simulink model "controller_observer_AllAgents_v5"  (Simulink 8.4)
settings: Solver=ode1  StartTime=0.0  StopTime=inf  FixedStep=60*1  RelTol=1e-3
values from neighbouring files (symbols this model uses):
  Cpw = 4180    [readepJSONout.m]
  m_max = 3    [DesignParameters.m]
  mc_MAX = 2    [DesignParameters.m]
  Tamb_additive_Sensor_fault_time = 60*60*24*(Tamb_day_ofthe_add_fault + Tamb_time_ofthe_add_fault)    (= 129600)    [faults_setting.m]
  Tamb_additive_Sensor_fault_value = 10    [faults_setting.m]
  Tamb_mult_Sensor_fault_time = 60*60*24*(Tamb_day_ofthe_mult_fault + Tamb_time_ofthe_mult_fault)    (= 129600)    [faults_setting.m]
  Tamb_mult_Sensor_fault_value = 1.1    [faults_setting.m]
  Ts = 3600/dat.Timestep.Timestep_0x20_1.number_of_timesteps_per_hour    [readepJSONout.m]
  Tst_C_additive_Sensor_fault_time = 60*60*24*(Tst_C_day_ofthe_add_fault + Tst_C_time_ofthe_add_fault)    (= 129600)    [faults_setting.m]
  Tst_C_additive_Sensor_fault_value = 10    [faults_setting.m]
  Tst_C_mult_Sensor_fault_time = 60*60*24*(Tst_C_day_ofthe_mult_fault + Tst_C_time_ofthe_mult_fault)    (= 129600)    [faults_setting.m]
  Tst_C_mult_Sensor_fault_value = 1.1    [faults_setting.m]
  Tst_H_additive_Sensor_fault_time = 60*60*24*(Tst_H_day_ofthe_add_fault + Tst_H_time_ofthe_add_fault)    (= 129600)    [faults_setting.m]
  Tst_H_additive_Sensor_fault_value = 10    [faults_setting.m]
  Tst_H_mult_Sensor_fault_time = 60*60*24*(Tst_H_day_ofthe_mult_fault + Tst_H_time_ofthe_mult_fault)    (= 129600)    [faults_setting.m]
  Tst_H_mult_Sensor_fault_value = 1.1    [faults_setting.m]
undefined symbols (not defined in the file or in its folder): building, COP, Ehp_max, fsa_E, fsa_ET, fsa_N, fsa_NT, fsa_W, fsa_WT, h_E, h_ET, h_N, h_NT, h_W, h_WT, K_NtoNT, K_NTtoN, Ke_E, Ke_ET, Ke_N, Ke_NT, Ke_WT, Km_E, Km_ET, Km_N, Km_NT, Km_W, Km_WT, Kout_N, Kout_NT, Kout_W, Kout_WT, Kst, Ksta, Kstcij, KstRef, Kw_E, Kw_ET, Kw_N, Kw_NT, Kw_W, Kw_WT
libraries: simulink x61
(part 10 of 36)

== system controller_observer_AllAgents_v5/Controller/CorridorPod3_Cooling ==
  In1 : Inport
  Enable : EnablePort
  Add : Sum  SampleTime=Ts
  Add1 : Sum  SampleTime=Ts
  CoilControlGains : Constant  Value=[building.zone(12).Kesa_C; building.zone(12).Ksa_C; building.zone(12).K_C; building.zone(12).Kma_C; building.zone(12).Kf_C]  SampleTime=Ts  FramePeriod=Ts
  Constant : Constant  Value=0.00001  SampleTime=Ts
  Constant1 : Constant  Value=0
  Demux : Demux  Outputs=[1 1 1 1 1 numel(building.zone(12).neighbour)]
  Discrete PID Controller : simulink/Continuous/PID Controller  Controller=PID  TimeDomain=Continuous-time  SampleTime=-1  IntegratorMethod=Forward Euler  FilterMethod=Forward Euler  Form=Parallel  P=1  I=1  D=0  N=100  InitialConditionSource=internal  InitialConditionForIntegrator=0  InitialConditionForFilter=0  ExternalReset=none  LimitOutput=off  UpperSaturationLimit=inf  LowerSaturationLimit=-inf  LinearizeAsGain=on  AntiWindupMode=none  Kb=1  TrackingMode=off  Kt=1  PParamMin=[]  PParamMax=[]  IParamMin=[]  IParamMax=[]  DParamMin=[]  DParamMax=[]  NParamMin=[]  NParamMax=[]  KbParamMin=[]  KbParamMax=[]  KtParamMin=[]  KtParamMax=[]  POutMin=[]  POutMax=[]  IOutMin=[]  IOutMax=[]  DOutMin=[]  DOutMax=[]  NOutMin=[]  NOutMax=[]  KbOutMin=[]  KbOutMax=[]  KtOutMin=[]  KtOutMax=[]  IntegratorOutMin=[]  IntegratorOutMax=[]  FilterOutMin=[]  FilterOutMax=[]  SumOutMin=[]  SumOutMax=[]  SumI1OutMin=[]  SumI1OutMax=[]  SumI2OutMin=[]  SumI2OutMax=[]  SumI3OutMin=[]  SumI3OutMax=[]  SumDOutMin=[]  SumDOutMax=[]  SaturationOutMin=[]  SaturationOutMax=[]  IntegratorContinuousStateAttributes=''  IntegratorStateMustResolveToSignalObject=off  IntegratorRTWStateStorageClass=Auto  FilterContinuousStateAttributes=''  FilterStateMustResolveToSignalObject=off  FilterRTWStateStorageClass=Auto  DifferentiatorICPrevScaledInput=0  DifferentiatorOutMin=[]  DifferentiatorOutMax=[]  UseFilter=on
  Divide : Product  Inputs=*/  SampleTime=Ts
  Divide1 : Product  Inputs=*/  SampleTime=Ts
  Dot Product : DotProduct  SampleTime=Ts  InputSameDT=on  OutDataTypeStr=Inherit: Inherit via internal rule
  Dot Product1 : DotProduct  SampleTime=Ts  InputSameDT=on  OutDataTypeStr=Inherit: Inherit via internal rule
  Dot Product2 : DotProduct  SampleTime=Ts  InputSameDT=on  OutDataTypeStr=Inherit: Inherit via internal rule
  Gain{,1..3} (4 alike) : Gain  Gain=-1  SampleTime=Ts
  If : If  IfExpression=u1 >= 0
  If Action Subsystem : Subsystem  TreatAsAtomicUnit=on
  If Action Subsystem1 : Subsystem  TreatAsAtomicUnit=on
  K_I_mat : ToFile  Filename=C:\Users\papad\Dropbox\Complete_building_simulator_Observers\Results_fz4_sensor\K_I_CorridorPod3.mat  SaveFormat=Timeseries
  MaxValveFlow : Constant  Value=m_max  SampleTime=Ts  FramePeriod=Ts
  Merge : Merge
  Mux : Mux  Inputs=3
  Mux1 : Mux  Inputs=2
  Mux2 : Mux  Inputs=5
  Mux3 : Mux  Inputs=5
      settings: DisplayOption=signals
  Product : Product  SampleTime=Ts
  Scope : Scope
  Scope1 : Scope
  Scope2 : Scope
  Subtract{,1..4} (5 alike) : Sum  Inputs=+-  SampleTime=Ts
  Tc_C_mat : ToFile  Filename=C:\Users\papad\Dropbox\Complete_building_simulator_Observers\Results_fz4_sensor\Tc_C_CorridorPod3.mat  SaveFormat=Timeseries
  Tc_C_ref_mat : ToFile  Filename=C:\Users\papad\Dropbox\Complete_building_simulator_Observers\Results_fz4_sensor\Tc_C_ref_CorridorPod3.mat  SaveFormat=Timeseries
  TsTc_err_C_mat : ToFile  Filename=C:\Users\papad\Dropbox\Complete_building_simulator_Observers\Results_fz4_sensor\TsTc_err_C_CorridorPod3.mat  SaveFormat=Timeseries
  Tsa_mat : ToFile  Filename=C:\Users\papad\Dropbox\Complete_building_simulator_Observers\Results_fz4_sensor\Tsa_CorridorPod3.mat  SaveFormat=Timeseries
  Tsa_ref_mat : ToFile  Filename=C:\Users\papad\Dropbox\Complete_building_simulator_Observers\Results_fz4_sensor\Tsa_ref_CorridorPod3.mat  SaveFormat=Timeseries
  Tst_Tc_C_mat : ToFile  Filename=C:\Users\papad\Dropbox\Complete_building_simulator_Observers\Results_fz4_sensor\Tst_Tc_C_CorridorPod3.mat  SaveFormat=Timeseries
  TzRef : Constant  Value=building.zone(12).TzRef  SampleTime=Ts  FramePeriod=Ts
  Tz_mat : ToFile  Filename=C:\Users\papad\Dropbox\Complete_building_simulator_Observers\Results_fz4_sensor\Tz_CorridorPod3.mat  SaveFormat=Timeseries
  Tz_ref_mat : ToFile  Filename=C:\Users\papad\Dropbox\Complete_building_simulator_Observers\Results_fz4_sensor\Tz_ref_CorridorPod3.mat  SaveFormat=Timeseries
  Unit Delay : UnitDelay  SampleTime=Ts
  ValveControlGains : Constant  Value=[building.zone(12).Kec_C; building.zone(12).Kc_C; building.zone(12).Kcsa_C]  SampleTime=Ts  FramePeriod=Ts
  ValveControlGains2 : Constant  SampleTime=Ts  FramePeriod=Ts
  ZoneControlGains : Constant  Value=[building.zone(12).Ke; building.zone(12).Kz; transpose(building.zone(12).Kij); building.zone(12).Kza; building.zone(12).hi]  SampleTime=Ts  FramePeriod=Ts
  er_Tz_mat : ToFile  Filename=C:\Users\papad\Dropbox\Complete_building_simulator_Observers\Results_fz4_sensor\er_Tz_CorridorPod3.mat  SaveFormat=Timeseries
  mc_C_mat : ToFile  Filename=C:\Users\papad\Dropbox\Complete_building_simulator_Observers\Results_fz4_sensor\mc_C_CorridorPod3.mat  SaveFormat=Timeseries
  mc_Tst_Tc_C_mat : ToFile  Filename=C:\Users\papad\Dropbox\Complete_building_simulator_Observers\Results_fz4_sensor\mc_Tst_Tc_C_CorridorPod3.mat  SaveFormat=Timeseries
  zone_gains_mat : ToFile  Filename=C:\Users\papad\Dropbox\Complete_building_simulator_Observers\Results_fz4_sensor\zone_gains_CorridorPod3.mat  SaveFormat=Timeseries
  Out1 : Outport
  Out2 : Outport  Port=2
  Out3 : Outport  Port=3
  wires:
  Mux/1 -> Dot Product/1
  CoilControlGains/1 -> Gain1/1
  Add/1 -> Unit Delay/1, K_I_mat/1, [K_I]
  Dot Product/1 -> Divide1/1, Scope/1, mc_Tst_Tc_C_mat/1, Divide/1
  Gain1/1 -> Dot Product1/2
  [TzRef] -> Subtract1/2
  ValveControlGains2/1 -> Mux2/5
  [Tst] -> Mux1/1, Subtract/1
  Mux2/1 -> Dot Product1/1
  Demux/6 -> [TzNeighbor]
  Demux/4 -> [Tsa]
  Mux3/1 -> Dot Product2/1
  Gain2/1 -> Dot Product2/2
  Subtract1/1 -> Add/1
  Dot Product2/1 -> Scope2/1, Tsa_ref_mat/1, [TsaRef], Subtract3/2, Mux2/2
  [Tc] -> Mux1/2, Subtract/2
  Dot Product1/1 -> Scope1/1, Tc_C_ref_mat/1, [TcRef], Mux/2, Subtract4/2
  [Tz] -> Subtract1/1
  Unit Delay/1 -> Add/2
  ZoneControlGains/1 -> zone_gains_mat/1, Gain2/1
  [Tsa] -> Mux/3
  [Tz] -> Mux2/3
  Demux/2 -> [Tamb]
  Demux/5 -> [Tst]
  Gain/1 -> Dot Product/2
  ValveControlGains/1 -> Gain/1
  In1/1 -> Demux/1
  [Tc] -> Add1/2
  Divide1/1 -> Add1/1
  MaxValveFlow/1 -> Divide1/2
  Add1/1 -> Out3/1, [TstRef]
  Product/1 -> Out2/1
  Gain3/1 -> Product/2
  Mux1/1 -> Tst_Tc_C_mat/1
  TzRef/1 -> [TzRef], Tz_ref_mat/1, Subtract2/2, Mux3/2
  Subtract3/1 -> Mux2/1
  [Tsa] -> Tsa_mat/1, Subtract3/1
  Subtract4/1 -> Mux/1
  [Tc] -> Subtract4/1, Tc_C_mat/1
  Constant1/1 -> If Action Subsystem/1(In1)
  If/1 -> If Action Subsystem/Action
  If/2 -> If Action Subsystem1/Action
  If Action Subsystem/1(Out1) -> Merge/1
  Subtract/1 -> TsTc_err_C_mat/1, Divide/2, If/1
  Divide/1 -> If Action Subsystem1/1(In1)
  If Action Subsystem1/1(Out1) -> Merge/2
  [Tz] -> Tz_mat/1, Subtract2/1
  [TzNeighbor] -> Mux3/3
  [Tamb] -> Mux2/4
  [Tamb] -> Mux3/4
  [K_I] -> Mux3/5
  Subtract2/1 -> Discrete PID Controller/1, er_Tz_mat/1, Mux3/1
  Demux/1 -> [Tz]
  Merge/1 -> mc_C_mat/1, Out1/1
  Demux/3 -> [Tc]

== system controller_observer_AllAgents_v5/Controller/CorridorPod3_Heating ==
  In1 : Inport
  Enable : EnablePort
  Add : Sum  SampleTime=Ts
  Add1 : Sum  SampleTime=Ts
  CoilControlGains : Constant  Value=[building.zone(12).Kesa_H; building.zone(12).Ksa_H; building.zone(12).K_H; building.zone(12).Kma_H; building.zone(12).Kf_H]  SampleTime=Ts  FramePeriod=Ts
  Constant : Constant  Value=0.00001  SampleTime=Ts
  Constant1 : Constant  Value=0
  Demux : Demux  Outputs=[1 1 1 1 1 numel(building.zone(12).neighbour)]
  Discrete PID Controller : simulink/Continuous/PID Controller  Controller=PID  TimeDomain=Continuous-time  SampleTime=-1  IntegratorMethod=Forward Euler  FilterMethod=Forward Euler  Form=Parallel  P=1  I=1  D=0  N=100  InitialConditionSource=internal  InitialConditionForIntegrator=0  InitialConditionForFilter=0  ExternalReset=none  LimitOutput=off  UpperSaturationLimit=inf  LowerSaturationLimit=-inf  LinearizeAsGain=on  AntiWindupMode=none  Kb=1  TrackingMode=off  Kt=1  PParamMin=[]  PParamMax=[]  IParamMin=[]  IParamMax=[]  DParamMin=[]  DParamMax=[]  NParamMin=[]  NParamMax=[]  KbParamMin=[]  KbParamMax=[]  KtParamMin=[]  KtParamMax=[]  POutMin=[]  POutMax=[]  IOutMin=[]  IOutMax=[]  DOutMin=[]  DOutMax=[]  NOutMin=[]  NOutMax=[]  KbOutMin=[]  KbOutMax=[]  KtOutMin=[]  KtOutMax=[]  IntegratorOutMin=[]  IntegratorOutMax=[]  FilterOutMin=[]  FilterOutMax=[]  SumOutMin=[]  SumOutMax=[]  SumI1OutMin=[]  SumI1OutMax=[]  SumI2OutMin=[]  SumI2OutMax=[]  SumI3OutMin=[]  SumI3OutMax=[]  SumDOutMin=[]  SumDOutMax=[]  SaturationOutMin=[]  SaturationOutMax=[]  IntegratorContinuousStateAttributes=''  IntegratorStateMustResolveToSignalObject=off  IntegratorRTWStateStorageClass=Auto  FilterContinuousStateAttributes=''  FilterStateMustResolveToSignalObject=off  FilterRTWStateStorageClass=Auto  DifferentiatorICPrevScaledInput=0  DifferentiatorOutMin=[]  DifferentiatorOutMax=[]  UseFilter=on
  Divide : Product  Inputs=*/  SampleTime=Ts
  Divide1 : Product  Inputs=*/  SampleTime=Ts
  Dot Product : DotProduct  SampleTime=Ts  InputSameDT=on  OutDataTypeStr=Inherit: Inherit via internal rule
  Dot Product1 : DotProduct  SampleTime=Ts  InputSameDT=on  OutDataTypeStr=Inherit: Inherit via internal rule
  Dot Product2 : DotProduct  SampleTime=Ts  InputSameDT=on  OutDataTypeStr=Inherit: Inherit via internal rule
  Gain{,1..3} (4 alike) : Gain  Gain=-1  SampleTime=Ts
  If : If  IfExpression=u1 <= 0
  If Action Subsystem : Subsystem  TreatAsAtomicUnit=on
  If Action Subsystem1 : Subsystem  TreatAsAtomicUnit=on
  MaxValveFlow : Constant  Value=m_max  SampleTime=Ts  FramePeriod=Ts
  Merge : Merge
  Mux : Mux  Inputs=3
  Mux1 : Mux  Inputs=2
  Mux2 : Mux  Inputs=5
  Mux3 : Mux  Inputs=5
      settings: DisplayOption=signals
  Product : Product  SampleTime=Ts
  Scope : Scope
  Scope1 : Scope
  Scope2 : Scope
  Subtract{,1..4} (5 alike) : Sum  Inputs=+-  SampleTime=Ts
  Tc_H_mat : ToFile  Filename=C:\Users\papad\Dropbox\Complete_building_simulator_Observers\Results_fz4_sensor\Tc_H_CorridorPod3.mat  SaveFormat=Timeseries
  Tc_H_ref_mat : ToFile  Filename=C:\Users\papad\Dropbox\Complete_building_simulator_Observers\Results_fz4_sensor\Tc_H_ref_CorridorPod3.mat  SaveFormat=Timeseries
  TsTc_err_H_mat : ToFile  Filename=C:\Users\papad\Dropbox\Complete_building_simulator_Observers\Results_fz4_sensor\TsTc_err_H_CorridorPod3.mat  SaveFormat=Timeseries
  Tst_Tc_H_mat : ToFile  Filename=C:\Users\papad\Dropbox\Complete_building_simulator_Observers\Results_fz4_sensor\Tst_Tc_H_CorridorPod3.mat  SaveFormat=Timeseries
  TzRef : Constant  Value=building.zone(12).TzRef  SampleTime=Ts  FramePeriod=Ts
  Unit Delay : UnitDelay  SampleTime=Ts
  ValveControlGains : Constant  Value=[building.zone(12).Kec_H; building.zone(12).Kc_H; building.zone(12).Kcsa_H]  SampleTime=Ts  FramePeriod=Ts
  ValveControlGains2 : Constant  SampleTime=Ts  FramePeriod=Ts
  ZoneControlGains : Constant  Value=[building.zone(12).Ke; building.zone(12).Kz; transpose(building.zone(12).Kij); building.zone(12).Kza; building.zone(12).hi]  SampleTime=Ts  FramePeriod=Ts
  mc_H_mat : ToFile  Filename=C:\Users\papad\Dropbox\Complete_building_simulator_Observers\Results_fz4_sensor\mc_H_CorridorPod3.mat  SaveFormat=Timeseries
  mc_Tst_Tc_H_mat : ToFile  Filename=C:\Users\papad\Dropbox\Complete_building_simulator_Observers\Results_fz4_sensor\mc_Tst_Tc_H_CorridorPod3.mat  SaveFormat=Timeseries
  Out1 : Outport
  Out2 : Outport  Port=2
  Out3 : Outport  Port=3
  wires:
  Mux/1 -> Dot Product/1
  CoilControlGains/1 -> Gain1/1
  Add/1 -> Unit Delay/1, [K_I]
  Dot Product/1 -> Divide1/1, Scope/1, mc_Tst_Tc_H_mat/1, Divide/1
  Gain1/1 -> Dot Product1/2
  [TzRef] -> Subtract1/2
  ValveControlGains2/1 -> Mux2/5
  [Tst] -> Mux1/1, Subtract/1
  Mux2/1 -> Dot Product1/1
  Demux/6 -> [TzNeighbor]
  Demux/4 -> [Tsa]
  Mux3/1 -> Dot Product2/1
  Gain2/1 -> Dot Product2/2
  Subtract1/1 -> Add/1
  Dot Product2/1 -> Scope2/1, [TsaRef], Subtract3/2, Mux2/2
  [Tc] -> Mux1/2, Subtract/2
  Dot Product1/1 -> Scope1/1, Tc_H_ref_mat/1, [TcRef], Mux/2, Subtract4/2
  [Tz] -> Subtract1/1
  Unit Delay/1 -> Add/2
  Demux/3 -> [Tc]
  ZoneControlGains/1 -> Gain2/1
  [Tsa] -> Mux/3
  [Tz] -> Mux2/3
  Demux/2 -> [Tamb]
  Demux/5 -> [Tst]
  Gain/1 -> Dot Product/2
  ValveControlGains/1 -> Gain/1
  In1/1 -> Demux/1
  [Tc] -> Add1/2
  Divide1/1 -> Add1/1
  MaxValveFlow/1 -> Divide1/2
  Add1/1 -> Out3/1, [TstRef]
  Product/1 -> Out2/1
  Gain3/1 -> Product/2
  Mux1/1 -> Tst_Tc_H_mat/1
  TzRef/1 -> [TzRef], Subtract2/2, Mux3/2
  Subtract2/1 -> Mux3/1
  Subtract3/1 -> Mux2/1
  [Tsa] -> Subtract3/1
  Subtract4/1 -> Mux/1
  [Tc] -> Subtract4/1, Tc_H_mat/1
  Constant1/1 -> If Action Subsystem/1(In1)
  If/1 -> If Action Subsystem/Action
  If/2 -> If Action Subsystem1/Action
  If Action Subsystem/1(Out1) -> Merge/1
  Subtract/1 -> TsTc_err_H_mat/1, Divide/2, If/1
  Divide/1 -> If Action Subsystem1/1(In1)
  If Action Subsystem1/1(Out1) -> Merge/2
  [Tz] -> Subtract2/1
  [TzNeighbor] -> Mux3/3
  [Tamb] -> Mux2/4
  [Tamb] -> Mux3/4
  [K_I] -> Mux3/5
  Demux/1 -> [Tz]
  Merge/1 -> Out1/1, mc_H_mat/1

== system controller_observer_AllAgents_v5/Controller/EnableDifonifmode1 ==
  mc : Inport
  on_off : Inport  Port=2
  Constant7 : Constant  SampleTime=Ts
  Constant8 : Constant  Value=0  SampleTime=Ts
  If Action Subsystem7 : Subsystem  TreatAsAtomicUnit=on
  If Action Subsystem8 : Subsystem  TreatAsAtomicUnit=on
  If5 : If  SampleTime=Ts
  Logical Operator : Logic  AllPortsSameDT=off  OutDataTypeStr=boolean  SampleTime=Ts
  Merge3 : Merge
  enable detection : Outport
  wires:
  mc/1 -> If5/1
  If Action Subsystem8/1(Out1) -> Merge3/2
  If5/2 -> If Action Subsystem8/Action
  If5/1 -> If Action Subsystem7/Action
  Constant8/1 -> If Action Subsystem8/1(In1)
  Constant7/1 -> If Action Subsystem7/1(In1)
  If Action Subsystem7/1(Out1) -> Merge3/1
  Logical Operator/1 -> enable detection/1
  Merge3/1 -> Logical Operator/1
  on_off/1 -> Logical Operator/2

== system controller_observer_AllAgents_v5/Controller/EnableDifonifmode10 ==
  mc : Inport
  on_off : Inport  Port=2
  Constant7 : Constant  SampleTime=Ts
  Constant8 : Constant  Value=0  SampleTime=Ts
  If Action Subsystem7 : Subsystem  TreatAsAtomicUnit=on
  If Action Subsystem8 : Subsystem  TreatAsAtomicUnit=on
  If5 : If  SampleTime=Ts
  Logical Operator : Logic  AllPortsSameDT=off  OutDataTypeStr=boolean  SampleTime=Ts
  Merge3 : Merge
  enable detection : Outport
  wires:
  mc/1 -> If5/1
  If Action Subsystem8/1(Out1) -> Merge3/2
  If5/2 -> If Action Subsystem8/Action
  If5/1 -> If Action Subsystem7/Action
  Constant8/1 -> If Action Subsystem8/1(In1)
  Constant7/1 -> If Action Subsystem7/1(In1)
  If Action Subsystem7/1(Out1) -> Merge3/1
  Logical Operator/1 -> enable detection/1
  Merge3/1 -> Logical Operator/1
  on_off/1 -> Logical Operator/2

== system controller_observer_AllAgents_v5/Controller/EnableDifonifmode11 ==
  mc : Inport
  on_off : Inport  Port=2
  Constant7 : Constant  SampleTime=Ts
  Constant8 : Constant  Value=0  SampleTime=Ts
  If Action Subsystem7 : Subsystem  TreatAsAtomicUnit=on
  If Action Subsystem8 : Subsystem  TreatAsAtomicUnit=on
  If5 : If  SampleTime=Ts
  Logical Operator : Logic  AllPortsSameDT=off  OutDataTypeStr=boolean  SampleTime=Ts
  Merge3 : Merge
  enable detection : Outport
  wires:
  mc/1 -> If5/1
  If Action Subsystem8/1(Out1) -> Merge3/2
  If5/2 -> If Action Subsystem8/Action
  If5/1 -> If Action Subsystem7/Action
  Constant8/1 -> If Action Subsystem8/1(In1)
  Constant7/1 -> If Action Subsystem7/1(In1)
  If Action Subsystem7/1(Out1) -> Merge3/1
  Logical Operator/1 -> enable detection/1
  Merge3/1 -> Logical Operator/1
  on_off/1 -> Logical Operator/2

== system controller_observer_AllAgents_v5/Controller/EnableDifonifmode12 ==
  mc : Inport
  on_off : Inport  Port=2
  Constant7 : Constant  SampleTime=Ts
  Constant8 : Constant  Value=0  SampleTime=Ts
  If Action Subsystem7 : Subsystem  TreatAsAtomicUnit=on
  If Action Subsystem8 : Subsystem  TreatAsAtomicUnit=on
  If5 : If  SampleTime=Ts
  Logical Operator : Logic  AllPortsSameDT=off  OutDataTypeStr=boolean  SampleTime=Ts
  Merge3 : Merge
  enable detection : Outport
  wires:
  mc/1 -> If5/1
  If Action Subsystem8/1(Out1) -> Merge3/2
  If5/2 -> If Action Subsystem8/Action
  If5/1 -> If Action Subsystem7/Action
  Constant8/1 -> If Action Subsystem8/1(In1)
  Constant7/1 -> If Action Subsystem7/1(In1)
  If Action Subsystem7/1(Out1) -> Merge3/1
  Logical Operator/1 -> enable detection/1
  Merge3/1 -> Logical Operator/1
  on_off/1 -> Logical Operator/2

== system controller_observer_AllAgents_v5/Controller/EnableDifonifmode13 ==
  mc : Inport
  on_off : Inport  Port=2
  Constant7 : Constant  SampleTime=Ts
  Constant8 : Constant  Value=0  SampleTime=Ts
  If Action Subsystem7 : Subsystem  TreatAsAtomicUnit=on
  If Action Subsystem8 : Subsystem  TreatAsAtomicUnit=on
  If5 : If  SampleTime=Ts
  Logical Operator : Logic  AllPortsSameDT=off  OutDataTypeStr=boolean  SampleTime=Ts
  Merge3 : Merge
  enable detection : Outport
  wires:
  mc/1 -> If5/1
  If Action Subsystem8/1(Out1) -> Merge3/2
  If5/2 -> If Action Subsystem8/Action
  If5/1 -> If Action Subsystem7/Action
  Constant8/1 -> If Action Subsystem8/1(In1)
  Constant7/1 -> If Action Subsystem7/1(In1)
  If Action Subsystem7/1(Out1) -> Merge3/1
  Logical Operator/1 -> enable detection/1
  Merge3/1 -> Logical Operator/1
  on_off/1 -> Logical Operator/2

== system controller_observer_AllAgents_v5/Controller/EnableDifonifmode14 ==
  mc : Inport
  on_off : Inport  Port=2
  Constant7 : Constant  SampleTime=Ts
  Constant8 : Constant  Value=0  SampleTime=Ts
  If Action Subsystem7 : Subsystem  TreatAsAtomicUnit=on
  If Action Subsystem8 : Subsystem  TreatAsAtomicUnit=on
  If5 : If  SampleTime=Ts
  Logical Operator : Logic  AllPortsSameDT=off  OutDataTypeStr=boolean  SampleTime=Ts
  Merge3 : Merge
  enable detection : Outport
  wires:
  mc/1 -> If5/1
  If Action Subsystem8/1(Out1) -> Merge3/2
  If5/2 -> If Action Subsystem8/Action
  If5/1 -> If Action Subsystem7/Action
  Constant8/1 -> If Action Subsystem8/1(In1)
  Constant7/1 -> If Action Subsystem7/1(In1)
  If Action Subsystem7/1(Out1) -> Merge3/1
  Logical Operator/1 -> enable detection/1
  Merge3/1 -> Logical Operator/1
  on_off/1 -> Logical Operator/2

== system controller_observer_AllAgents_v5/Controller/EnableDifonifmode15 ==
  mc : Inport
  on_off : Inport  Port=2
  Constant7 : Constant  SampleTime=Ts
  Constant8 : Constant  Value=0  SampleTime=Ts
  If Action Subsystem7 : Subsystem  TreatAsAtomicUnit=on
  If Action Subsystem8 : Subsystem  TreatAsAtomicUnit=on
  If5 : If  SampleTime=Ts
  Logical Operator : Logic  AllPortsSameDT=off  OutDataTypeStr=boolean  SampleTime=Ts
  Merge3 : Merge
  enable detection : Outport
  wires:
  mc/1 -> If5/1
  If Action Subsystem8/1(Out1) -> Merge3/2
  If5/2 -> If Action Subsystem8/Action
  If5/1 -> If Action Subsystem7/Action
  Constant8/1 -> If Action Subsystem8/1(In1)
  Constant7/1 -> If Action Subsystem7/1(In1)
  If Action Subsystem7/1(Out1) -> Merge3/1
  Logical Operator/1 -> enable detection/1
  Merge3/1 -> Logical Operator/1
  on_off/1 -> Logical Operator/2

== system controller_observer_AllAgents_v5/Controller/EnableDifonifmode16 ==
  mc : Inport
  on_off : Inport  Port=2
  Constant7 : Constant  SampleTime=Ts
  Constant8 : Constant  Value=0  SampleTime=Ts
  If Action Subsystem7 : Subsystem  TreatAsAtomicUnit=on
  If Action Subsystem8 : Subsystem  TreatAsAtomicUnit=on
  If5 : If  SampleTime=Ts
  Logical Operator : Logic  AllPortsSameDT=off  OutDataTypeStr=boolean  SampleTime=Ts
  Merge3 : Merge
  enable detection : Outport
  wires:
  mc/1 -> If5/1
  If Action Subsystem8/1(Out1) -> Merge3/2
  If5/2 -> If Action Subsystem8/Action
  If5/1 -> If Action Subsystem7/Action
  Constant8/1 -> If Action Subsystem8/1(In1)
  Constant7/1 -> If Action Subsystem7/1(In1)
  If Action Subsystem7/1(Out1) -> Merge3/1
  Logical Operator/1 -> enable detection/1
  Merge3/1 -> Logical Operator/1
  on_off/1 -> Logical Operator/2

== system controller_observer_AllAgents_v5/Controller/EnableDifonifmode17 ==
  mc : Inport
  on_off : Inport  Port=2
  Constant7 : Constant  SampleTime=Ts
  Constant8 : Constant  Value=0  SampleTime=Ts
  If Action Subsystem7 : Subsystem  TreatAsAtomicUnit=on
  If Action Subsystem8 : Subsystem  TreatAsAtomicUnit=on
  If5 : If  SampleTime=Ts
  Logical Operator : Logic  AllPortsSameDT=off  OutDataTypeStr=boolean  SampleTime=Ts
  Merge3 : Merge
  enable detection : Outport
  wires:
  mc/1 -> If5/1
  If Action Subsystem8/1(Out1) -> Merge3/2
  If5/2 -> If Action Subsystem8/Action
  If5/1 -> If Action Subsystem7/Action
  Constant8/1 -> If Action Subsystem8/1(In1)
  Constant7/1 -> If Action Subsystem7/1(In1)
  If Action Subsystem7/1(Out1) -> Merge3/1
  Logical Operator/1 -> enable detection/1
  Merge3/1 -> Logical Operator/1
  on_off/1 -> Logical Operator/2

== system controller_observer_AllAgents_v5/Controller/EnableDifonifmode18 ==
  mc : Inport
  on_off : Inport  Port=2
  Constant7 : Constant  SampleTime=Ts
  Constant8 : Constant  Value=0  SampleTime=Ts
  If Action Subsystem7 : Subsystem  TreatAsAtomicUnit=on
  If Action Subsystem8 : Subsystem  TreatAsAtomicUnit=on
  If5 : If  SampleTime=Ts
  Logical Operator : Logic  AllPortsSameDT=off  OutDataTypeStr=boolean  SampleTime=Ts
  Merge3 : Merge
  enable detection : Outport
  wires:
  mc/1 -> If5/1
  If Action Subsystem8/1(Out1) -> Merge3/2
  If5/2 -> If Action Subsystem8/Action
  If5/1 -> If Action Subsystem7/Action
  Constant8/1 -> If Action Subsystem8/1(In1)
  Constant7/1 -> If Action Subsystem7/1(In1)
  If Action Subsystem7/1(Out1) -> Merge3/1
  Logical Operator/1 -> enable detection/1
  Merge3/1 -> Logical Operator/1
  on_off/1 -> Logical Operator/2

== system controller_observer_AllAgents_v5/Controller/EnableDifonifmode19 ==
  mc : Inport
  on_off : Inport  Port=2
  Constant7 : Constant  SampleTime=Ts
  Constant8 : Constant  Value=0  SampleTime=Ts
  If Action Subsystem7 : Subsystem  TreatAsAtomicUnit=on
  If Action Subsystem8 : Subsystem  TreatAsAtomicUnit=on
  If5 : If  SampleTime=Ts
  Logical Operator : Logic  AllPortsSameDT=off  OutDataTypeStr=boolean  SampleTime=Ts
  Merge3 : Merge
  enable detection : Outport
  wires:
  mc/1 -> If5/1
  If Action Subsystem8/1(Out1) -> Merge3/2
  If5/2 -> If Action Subsystem8/Action
  If5/1 -> If Action Subsystem7/Action
  Constant8/1 -> If Action Subsystem8/1(In1)
  Constant7/1 -> If Action Subsystem7/1(In1)
  If Action Subsystem7/1(Out1) -> Merge3/1
  Logical Operator/1 -> enable detection/1
  Merge3/1 -> Logical Operator/1
  on_off/1 -> Logical Operator/2

== system controller_observer_AllAgents_v5/Controller/EnableDifonifmode2 ==
  mc : Inport
  on_off : Inport  Port=2
  Constant7 : Constant  SampleTime=Ts
  Constant8 : Constant  Value=0  SampleTime=Ts
  If Action Subsystem7 : Subsystem  TreatAsAtomicUnit=on
  If Action Subsystem8 : Subsystem  TreatAsAtomicUnit=on
  If5 : If  SampleTime=Ts
  Logical Operator : Logic  AllPortsSameDT=off  OutDataTypeStr=boolean  SampleTime=Ts
  Merge3 : Merge
  enable detection : Outport
  wires:
  mc/1 -> If5/1
  If Action Subsystem8/1(Out1) -> Merge3/2
  If5/2 -> If Action Subsystem8/Action
  If5/1 -> If Action Subsystem7/Action
  Constant8/1 -> If Action Subsystem8/1(In1)
  Constant7/1 -> If Action Subsystem7/1(In1)
  If Action Subsystem7/1(Out1) -> Merge3/1
  Logical Operator/1 -> enable detection/1
  Merge3/1 -> Logical Operator/1
  on_off/1 -> Logical Operator/2

== system controller_observer_AllAgents_v5/Controller/EnableDifonifmode20 ==
  mc : Inport
  on_off : Inport  Port=2
  Constant7 : Constant  SampleTime=Ts
  Constant8 : Constant  Value=0  SampleTime=Ts
  If Action Subsystem7 : Subsystem  TreatAsAtomicUnit=on
  If Action Subsystem8 : Subsystem  TreatAsAtomicUnit=on
  If5 : If  SampleTime=Ts
  Logical Operator : Logic  AllPortsSameDT=off  OutDataTypeStr=boolean  SampleTime=Ts
  Merge3 : Merge
  enable detection : Outport
  wires:
  mc/1 -> If5/1
  If Action Subsystem8/1(Out1) -> Merge3/2
  If5/2 -> If Action Subsystem8/Action
  If5/1 -> If Action Subsystem7/Action
  Constant8/1 -> If Action Subsystem8/1(In1)
  Constant7/1 -> If Action Subsystem7/1(In1)
  If Action Subsystem7/1(Out1) -> Merge3/1
  Logical Operator/1 -> enable detection/1
  Merge3/1 -> Logical Operator/1
  on_off/1 -> Logical Operator/2

== system controller_observer_AllAgents_v5/Controller/EnableDifonifmode21 ==
  mc : Inport
  on_off : Inport  Port=2
  Constant7 : Constant  SampleTime=Ts
  Constant8 : Constant  Value=0  SampleTime=Ts
  If Action Subsystem7 : Subsystem  TreatAsAtomicUnit=on
  If Action Subsystem8 : Subsystem  TreatAsAtomicUnit=on
  If5 : If  SampleTime=Ts
  Logical Operator : Logic  AllPortsSameDT=off  OutDataTypeStr=boolean  SampleTime=Ts
  Merge3 : Merge
  enable detection : Outport
  wires:
  mc/1 -> If5/1
  If Action Subsystem8/1(Out1) -> Merge3/2
  If5/2 -> If Action Subsystem8/Action
  If5/1 -> If Action Subsystem7/Action
  Constant8/1 -> If Action Subsystem8/1(In1)
  Constant7/1 -> If Action Subsystem7/1(In1)
  If Action Subsystem7/1(Out1) -> Merge3/1
  Logical Operator/1 -> enable detection/1
  Merge3/1 -> Logical Operator/1
  on_off/1 -> Logical Operator/2

== system controller_observer_AllAgents_v5/Controller/EnableDifonifmode22 ==
  mc : Inport
  on_off : Inport  Port=2
  Constant7 : Constant  SampleTime=Ts
  Constant8 : Constant  Value=0  SampleTime=Ts
  If Action Subsystem7 : Subsystem  TreatAsAtomicUnit=on
  If Action Subsystem8 : Subsystem  TreatAsAtomicUnit=on
  If5 : If  SampleTime=Ts
  Logical Operator : Logic  AllPortsSameDT=off  OutDataTypeStr=boolean  SampleTime=Ts
  Merge3 : Merge
  enable detection : Outport
  wires:
  mc/1 -> If5/1
  If Action Subsystem8/1(Out1) -> Merge3/2
  If5/2 -> If Action Subsystem8/Action
  If5/1 -> If Action Subsystem7/Action
  Constant8/1 -> If Action Subsystem8/1(In1)
  Constant7/1 -> If Action Subsystem7/1(In1)
  If Action Subsystem7/1(Out1) -> Merge3/1
  Logical Operator/1 -> enable detection/1
  Merge3/1 -> Logical Operator/1
  on_off/1 -> Logical Operator/2

== system controller_observer_AllAgents_v5/Controller/EnableDifonifmode23 ==
  mc : Inport
  on_off : Inport  Port=2
  Constant7 : Constant  SampleTime=Ts
  Constant8 : Constant  Value=0  SampleTime=Ts
  If Action Subsystem7 : Subsystem  TreatAsAtomicUnit=on
  If Action Subsystem8 : Subsystem  TreatAsAtomicUnit=on
  If5 : If  SampleTime=Ts
  Logical Operator : Logic  AllPortsSameDT=off  OutDataTypeStr=boolean  SampleTime=Ts
  Merge3 : Merge
  enable detection : Outport
  wires:
  mc/1 -> If5/1
  If Action Subsystem8/1(Out1) -> Merge3/2
  If5/2 -> If Action Subsystem8/Action
  If5/1 -> If Action Subsystem7/Action
  Constant8/1 -> If Action Subsystem8/1(In1)
  Constant7/1 -> If Action Subsystem7/1(In1)
  If Action Subsystem7/1(Out1) -> Merge3/1
  Logical Operator/1 -> enable detection/1
  Merge3/1 -> Logical Operator/1
  on_off/1 -> Logical Operator/2

== system controller_observer_AllAgents_v5/Controller/EnableDifonifmode24 ==
  mc : Inport
  on_off : Inport  Port=2
  Constant7 : Constant  SampleTime=Ts
  Constant8 : Constant  Value=0  SampleTime=Ts
  If Action Subsystem7 : Subsystem  TreatAsAtomicUnit=on
  If Action Subsystem8 : Subsystem  TreatAsAtomicUnit=on
  If5 : If  SampleTime=Ts
  Logical Operator : Logic  AllPortsSameDT=off  OutDataTypeStr=boolean  SampleTime=Ts
  Merge3 : Merge
  enable detection : Outport
  wires:
  mc/1 -> If5/1
  If Action Subsystem8/1(Out1) -> Merge3/2
  If5/2 -> If Action Subsystem8/Action
  If5/1 -> If Action Subsystem7/Action
  Constant8/1 -> If Action Subsystem8/1(In1)
  Constant7/1 -> If Action Subsystem7/1(In1)
  If Action Subsystem7/1(Out1) -> Merge3/1
  Logical Operator/1 -> enable detection/1
  Merge3/1 -> Logical Operator/1
  on_off/1 -> Logical Operator/2

== system controller_observer_AllAgents_v5/Controller/EnableDifonifmode25 ==
  mc : Inport
  on_off : Inport  Port=2
  Constant7 : Constant  SampleTime=Ts
  Constant8 : Constant  Value=0  SampleTime=Ts
  If Action Subsystem7 : Subsystem  TreatAsAtomicUnit=on
  If Action Subsystem8 : Subsystem  TreatAsAtomicUnit=on
  If5 : If  SampleTime=Ts
  Logical Operator : Logic  AllPortsSameDT=off  OutDataTypeStr=boolean  SampleTime=Ts
  Merge3 : Merge
  enable detection : Outport
  wires:
  mc/1 -> If5/1
  If Action Subsystem8/1(Out1) -> Merge3/2
  If5/2 -> If Action Subsystem8/Action
  If5/1 -> If Action Subsystem7/Action
  Constant8/1 -> If Action Subsystem8/1(In1)
  Constant7/1 -> If Action Subsystem7/1(In1)
  If Action Subsystem7/1(Out1) -> Merge3/1
  Logical Operator/1 -> enable detection/1
  Merge3/1 -> Logical Operator/1
  on_off/1 -> Logical Operator/2

== system controller_observer_AllAgents_v5/Controller/EnableDifonifmode26 ==
  mc : Inport
  on_off : Inport  Port=2
  Constant7 : Constant  SampleTime=Ts
  Constant8 : Constant  Value=0  SampleTime=Ts
  If Action Subsystem7 : Subsystem  TreatAsAtomicUnit=on
  If Action Subsystem8 : Subsystem  TreatAsAtomicUnit=on
  If5 : If  SampleTime=Ts
  Logical Operator : Logic  AllPortsSameDT=off  OutDataTypeStr=boolean  SampleTime=Ts
  Merge3 : Merge
  enable detection : Outport
  wires:
  mc/1 -> If5/1
  If Action Subsystem8/1(Out1) -> Merge3/2
  If5/2 -> If Action Subsystem8/Action
  If5/1 -> If Action Subsystem7/Action
  Constant8/1 -> If Action Subsystem8/1(In1)
  Constant7/1 -> If Action Subsystem7/1(In1)
  If Action Subsystem7/1(Out1) -> Merge3/1
  Logical Operator/1 -> enable detection/1
  Merge3/1 -> Logical Operator/1
  on_off/1 -> Logical Operator/2

== system controller_observer_AllAgents_v5/Controller/EnableDifonifmode27 ==
  mc : Inport
  on_off : Inport  Port=2
  Constant7 : Constant  SampleTime=Ts
  Constant8 : Constant  Value=0  SampleTime=Ts
  If Action Subsystem7 : Subsystem  TreatAsAtomicUnit=on
  If Action Subsystem8 : Subsystem  TreatAsAtomicUnit=on
  If5 : If  SampleTime=Ts
  Logical Operator : Logic  AllPortsSameDT=off  OutDataTypeStr=boolean  SampleTime=Ts
  Merge3 : Merge
  enable detection : Outport
  wires:
  mc/1 -> If5/1
  If Action Subsystem8/1(Out1) -> Merge3/2
  If5/2 -> If Action Subsystem8/Action
  If5/1 -> If Action Subsystem7/Action
  Constant8/1 -> If Action Subsystem8/1(In1)
  Constant7/1 -> If Action Subsystem7/1(In1)
  If Action Subsystem7/1(Out1) -> Merge3/1
  Logical Operator/1 -> enable detection/1
  Merge3/1 -> Logical Operator/1
  on_off/1 -> Logical Operator/2

== system controller_observer_AllAgents_v5/Controller/EnableDifonifmode28 ==
  mc : Inport
  on_off : Inport  Port=2
  Constant7 : Constant  SampleTime=Ts
  Constant8 : Constant  Value=0  SampleTime=Ts
  If Action Subsystem7 : Subsystem  TreatAsAtomicUnit=on
  If Action Subsystem8 : Subsystem  TreatAsAtomicUnit=on
  If5 : If  SampleTime=Ts
  Logical Operator : Logic  AllPortsSameDT=off  OutDataTypeStr=boolean  SampleTime=Ts
  Merge3 : Merge
  enable detection : Outport
  wires:
  mc/1 -> If5/1
  If Action Subsystem8/1(Out1) -> Merge3/2
  If5/2 -> If Action Subsystem8/Action
  If5/1 -> If Action Subsystem7/Action
  Constant8/1 -> If Action Subsystem8/1(In1)
  Constant7/1 -> If Action Subsystem7/1(In1)
  If Action Subsystem7/1(Out1) -> Merge3/1
  Logical Operator/1 -> enable detection/1
  Merge3/1 -> Logical Operator/1
  on_off/1 -> Logical Operator/2

== system controller_observer_AllAgents_v5/Controller/EnableDifonifmode29 ==
  mc : Inport
  on_off : Inport  Port=2
  Constant7 : Constant  SampleTime=Ts
  Constant8 : Constant  Value=0  SampleTime=Ts
  If Action Subsystem7 : Subsystem  TreatAsAtomicUnit=on
  If Action Subsystem8 : Subsystem  TreatAsAtomicUnit=on
  If5 : If  SampleTime=Ts
  Logical Operator : Logic  AllPortsSameDT=off  OutDataTypeStr=boolean  SampleTime=Ts
  Merge3 : Merge
  enable detection : Outport
  wires:
  mc/1 -> If5/1
  If Action Subsystem8/1(Out1) -> Merge3/2
  If5/2 -> If Action Subsystem8/Action
  If5/1 -> If Action Subsystem7/Action
  Constant8/1 -> If Action Subsystem8/1(In1)
  Constant7/1 -> If Action Subsystem7/1(In1)
  If Action Subsystem7/1(Out1) -> Merge3/1
  Logical Operator/1 -> enable detection/1
  Merge3/1 -> Logical Operator/1
  on_off/1 -> Logical Operator/2

== system controller_observer_AllAgents_v5/Controller/EnableDifonifmode3 ==
  mc : Inport
  on_off : Inport  Port=2
  Constant7 : Constant  SampleTime=Ts
  Constant8 : Constant  Value=0  SampleTime=Ts
  If Action Subsystem7 : Subsystem  TreatAsAtomicUnit=on
  If Action Subsystem8 : Subsystem  TreatAsAtomicUnit=on
  If5 : If  SampleTime=Ts
  Logical Operator : Logic  AllPortsSameDT=off  OutDataTypeStr=boolean  SampleTime=Ts
  Merge3 : Merge
  enable detection : Outport
  wires:
  mc/1 -> If5/1
  If Action Subsystem8/1(Out1) -> Merge3/2
  If5/2 -> If Action Subsystem8/Action
  If5/1 -> If Action Subsystem7/Action
  Constant8/1 -> If Action Subsystem8/1(In1)
  Constant7/1 -> If Action Subsystem7/1(In1)
  If Action Subsystem7/1(Out1) -> Merge3/1
  Logical Operator/1 -> enable detection/1
  Merge3/1 -> Logical Operator/1
  on_off/1 -> Logical Operator/2

== system controller_observer_AllAgents_v5/Controller/EnableDifonifmode30 ==
  mc : Inport
  on_off : Inport  Port=2
  Constant7 : Constant  SampleTime=Ts
  Constant8 : Constant  Value=0  SampleTime=Ts
  If Action Subsystem7 : Subsystem  TreatAsAtomicUnit=on
  If Action Subsystem8 : Subsystem  TreatAsAtomicUnit=on
  If5 : If  SampleTime=Ts
  Logical Operator : Logic  AllPortsSameDT=off  OutDataTypeStr=boolean  SampleTime=Ts
  Merge3 : Merge
  enable detection : Outport
  wires:
  mc/1 -> If5/1
  If Action Subsystem8/1(Out1) -> Merge3/2
  If5/2 -> If Action Subsystem8/Action
  If5/1 -> If Action Subsystem7/Action
  Constant8/1 -> If Action Subsystem8/1(In1)
  Constant7/1 -> If Action Subsystem7/1(In1)
  If Action Subsystem7/1(Out1) -> Merge3/1
  Logical Operator/1 -> enable detection/1
  Merge3/1 -> Logical Operator/1
  on_off/1 -> Logical Operator/2

== system controller_observer_AllAgents_v5/Controller/EnableDifonifmode31 ==
  mc : Inport
  on_off : Inport  Port=2
  Constant7 : Constant  SampleTime=Ts
  Constant8 : Constant  Value=0  SampleTime=Ts
  If Action Subsystem7 : Subsystem  TreatAsAtomicUnit=on
  If Action Subsystem8 : Subsystem  TreatAsAtomicUnit=on
  If5 : If  SampleTime=Ts
  Logical Operator : Logic  AllPortsSameDT=off  OutDataTypeStr=boolean  SampleTime=Ts
  Merge3 : Merge
  enable detection : Outport
  wires:
  mc/1 -> If5/1
  If Action Subsystem8/1(Out1) -> Merge3/2
  If5/2 -> If Action Subsystem8/Action
  If5/1 -> If Action Subsystem7/Action
  Constant8/1 -> If Action Subsystem8/1(In1)
  Constant7/1 -> If Action Subsystem7/1(In1)
  If Action Subsystem7/1(Out1) -> Merge3/1
  Logical Operator/1 -> enable detection/1
  Merge3/1 -> Logical Operator/1
  on_off/1 -> Logical Operator/2

== system controller_observer_AllAgents_v5/Controller/EnableDifonifmode32 ==
  mc : Inport
  on_off : Inport  Port=2
  Constant7 : Constant  SampleTime=Ts
  Constant8 : Constant  Value=0  SampleTime=Ts
  If Action Subsystem7 : Subsystem  TreatAsAtomicUnit=on
  If Action Subsystem8 : Subsystem  TreatAsAtomicUnit=on
  If5 : If  SampleTime=Ts
  Logical Operator : Logic  AllPortsSameDT=off  OutDataTypeStr=boolean  SampleTime=Ts
  Merge3 : Merge
  enable detection : Outport
  wires:
  mc/1 -> If5/1
  If Action Subsystem8/1(Out1) -> Merge3/2
  If5/2 -> If Action Subsystem8/Action
  If5/1 -> If Action Subsystem7/Action
  Constant8/1 -> If Action Subsystem8/1(In1)
  Constant7/1 -> If Action Subsystem7/1(In1)
  If Action Subsystem7/1(Out1) -> Merge3/1
  Logical Operator/1 -> enable detection/1
  Merge3/1 -> Logical Operator/1
  on_off/1 -> Logical Operator/2

== system controller_observer_AllAgents_v5/Controller/EnableDifonifmode33 ==
  mc : Inport
  on_off : Inport  Port=2
  Constant7 : Constant  SampleTime=Ts
  Constant8 : Constant  Value=0  SampleTime=Ts
  If Action Subsystem7 : Subsystem  TreatAsAtomicUnit=on
  If Action Subsystem8 : Subsystem  TreatAsAtomicUnit=on
  If5 : If  SampleTime=Ts
  Logical Operator : Logic  AllPortsSameDT=off  OutDataTypeStr=boolean  SampleTime=Ts
  Merge3 : Merge
  enable detection : Outport
  wires:
  mc/1 -> If5/1
  If Action Subsystem8/1(Out1) -> Merge3/2
  If5/2 -> If Action Subsystem8/Action
  If5/1 -> If Action Subsystem7/Action
  Constant8/1 -> If Action Subsystem8/1(In1)
  Constant7/1 -> If Action Subsystem7/1(In1)
  If Action Subsystem7/1(Out1) -> Merge3/1
  Logical Operator/1 -> enable detection/1
  Merge3/1 -> Logical Operator/1
  on_off/1 -> Logical Operator/2

== system controller_observer_AllAgents_v5/Controller/EnableDifonifmode34 ==
  mc : Inport
  on_off : Inport  Port=2
  Constant7 : Constant  SampleTime=Ts
  Constant8 : Constant  Value=0  SampleTime=Ts
  If Action Subsystem7 : Subsystem  TreatAsAtomicUnit=on
  If Action Subsystem8 : Subsystem  TreatAsAtomicUnit=on
  If5 : If  SampleTime=Ts
  Logical Operator : Logic  AllPortsSameDT=off  OutDataTypeStr=boolean  SampleTime=Ts
  Merge3 : Merge
  enable detection : Outport
  wires:
  mc/1 -> If5/1
  If Action Subsystem8/1(Out1) -> Merge3/2
  If5/2 -> If Action Subsystem8/Action
  If5/1 -> If Action Subsystem7/Action
  Constant8/1 -> If Action Subsystem8/1(In1)
  Constant7/1 -> If Action Subsystem7/1(In1)
  If Action Subsystem7/1(Out1) -> Merge3/1
  Logical Operator/1 -> enable detection/1
  Merge3/1 -> Logical Operator/1
  on_off/1 -> Logical Operator/2

== system controller_observer_AllAgents_v5/Controller/EnableDifonifmode35 ==
  mc : Inport
  on_off : Inport  Port=2
  Constant7 : Constant  SampleTime=Ts
  Constant8 : Constant  Value=0  SampleTime=Ts
  If Action Subsystem7 : Subsystem  TreatAsAtomicUnit=on
  If Action Subsystem8 : Subsystem  TreatAsAtomicUnit=on
  If5 : If  SampleTime=Ts
  Logical Operator : Logic  AllPortsSameDT=off  OutDataTypeStr=boolean  SampleTime=Ts
  Merge3 : Merge
  enable detection : Outport
  wires:
  mc/1 -> If5/1
  If Action Subsystem8/1(Out1) -> Merge3/2
  If5/2 -> If Action Subsystem8/Action
  If5/1 -> If Action Subsystem7/Action
  Constant8/1 -> If Action Subsystem8/1(In1)
  Constant7/1 -> If Action Subsystem7/1(In1)
  If Action Subsystem7/1(Out1) -> Merge3/1
  Logical Operator/1 -> enable detection/1
  Merge3/1 -> Logical Operator/1
  on_off/1 -> Logical Operator/2

== system controller_observer_AllAgents_v5/Controller/EnableDifonifmode36 ==
  mc : Inport
  on_off : Inport  Port=2
  Constant7 : Constant  SampleTime=Ts
  Constant8 : Constant  Value=0  SampleTime=Ts
  If Action Subsystem7 : Subsystem  TreatAsAtomicUnit=on
  If Action Subsystem8 : Subsystem  TreatAsAtomicUnit=on
  If5 : If  SampleTime=Ts
  Logical Operator : Logic  AllPortsSameDT=off  OutDataTypeStr=boolean  SampleTime=Ts
  Merge3 : Merge
  enable detection : Outport
  wires:
  mc/1 -> If5/1
  If Action Subsystem8/1(Out1) -> Merge3/2
  If5/2 -> If Action Subsystem8/Action
  If5/1 -> If Action Subsystem7/Action
  Constant8/1 -> If Action Subsystem8/1(In1)
  Constant7/1 -> If Action Subsystem7/1(In1)
  If Action Subsystem7/1(Out1) -> Merge3/1
  Logical Operator/1 -> enable detection/1
  Merge3/1 -> Logical Operator/1
  on_off/1 -> Logical Operator/2

== system controller_observer_AllAgents_v5/Controller/EnableDifonifmode37 ==
  mc : Inport
  on_off : Inport  Port=2
  Constant7 : Constant  SampleTime=Ts
  Constant8 : Constant  Value=0  SampleTime=Ts
  If Action Subsystem7 : Subsystem  TreatAsAtomicUnit=on
  If Action Subsystem8 : Subsystem  TreatAsAtomicUnit=on
  If5 : If  SampleTime=Ts
  Logical Operator : Logic  AllPortsSameDT=off  OutDataTypeStr=boolean  SampleTime=Ts
  Merge3 : Merge
  enable detection : Outport
  wires:
  mc/1 -> If5/1
  If Action Subsystem8/1(Out1) -> Merge3/2
  If5/2 -> If Action Subsystem8/Action
  If5/1 -> If Action Subsystem7/Action
  Constant8/1 -> If Action Subsystem8/1(In1)
  Constant7/1 -> If Action Subsystem7/1(In1)
  If Action Subsystem7/1(Out1) -> Merge3/1
  Logical Operator/1 -> enable detection/1
  Merge3/1 -> Logical Operator/1
  on_off/1 -> Logical Operator/2

== system controller_observer_AllAgents_v5/Controller/EnableDifonifmode38 ==
  mc : Inport
  on_off : Inport  Port=2
  Constant7 : Constant  SampleTime=Ts
  Constant8 : Constant  Value=0  SampleTime=Ts
  If Action Subsystem7 : Subsystem  TreatAsAtomicUnit=on
  If Action Subsystem8 : Subsystem  TreatAsAtomicUnit=on
  If5 : If  SampleTime=Ts
  Logical Operator : Logic  AllPortsSameDT=off  OutDataTypeStr=boolean  SampleTime=Ts
  Merge3 : Merge
  enable detection : Outport
  wires:
  mc/1 -> If5/1
  If Action Subsystem8/1(Out1) -> Merge3/2
  If5/2 -> If Action Subsystem8/Action
  If5/1 -> If Action Subsystem7/Action
  Constant8/1 -> If Action Subsystem8/1(In1)
  Constant7/1 -> If Action Subsystem7/1(In1)
  If Action Subsystem7/1(Out1) -> Merge3/1
  Logical Operator/1 -> enable detection/1
  Merge3/1 -> Logical Operator/1
  on_off/1 -> Logical Operator/2

== system controller_observer_AllAgents_v5/Controller/EnableDifonifmode39 ==
  mc : Inport
  on_off : Inport  Port=2
  Constant7 : Constant  SampleTime=Ts
  Constant8 : Constant  Value=0  SampleTime=Ts
  If Action Subsystem7 : Subsystem  TreatAsAtomicUnit=on
  If Action Subsystem8 : Subsystem  TreatAsAtomicUnit=on
  If5 : If  SampleTime=Ts
  Logical Operator : Logic  AllPortsSameDT=off  OutDataTypeStr=boolean  SampleTime=Ts
  Merge3 : Merge
  enable detection : Outport
  wires:
  mc/1 -> If5/1
  If Action Subsystem8/1(Out1) -> Merge3/2
  If5/2 -> If Action Subsystem8/Action
  If5/1 -> If Action Subsystem7/Action
  Constant8/1 -> If Action Subsystem8/1(In1)
  Constant7/1 -> If Action Subsystem7/1(In1)
  If Action Subsystem7/1(Out1) -> Merge3/1
  Logical Operator/1 -> enable detection/1
  Merge3/1 -> Logical Operator/1
  on_off/1 -> Logical Operator/2

== system controller_observer_AllAgents_v5/Controller/EnableDifonifmode4 ==
  mc : Inport
  on_off : Inport  Port=2
  Constant7 : Constant  SampleTime=Ts
  Constant8 : Constant  Value=0  SampleTime=Ts
  If Action Subsystem7 : Subsystem  TreatAsAtomicUnit=on
  If Action Subsystem8 : Subsystem  TreatAsAtomicUnit=on
  If5 : If  SampleTime=Ts
  Logical Operator : Logic  AllPortsSameDT=off  OutDataTypeStr=boolean  SampleTime=Ts
  Merge3 : Merge
  enable detection : Outport
  wires:
  mc/1 -> If5/1
  If Action Subsystem8/1(Out1) -> Merge3/2
  If5/2 -> If Action Subsystem8/Action
  If5/1 -> If Action Subsystem7/Action
  Constant8/1 -> If Action Subsystem8/1(In1)
  Constant7/1 -> If Action Subsystem7/1(In1)
  If Action Subsystem7/1(Out1) -> Merge3/1
  Logical Operator/1 -> enable detection/1
  Merge3/1 -> Logical Operator/1
  on_off/1 -> Logical Operator/2

== system controller_observer_AllAgents_v5/Controller/EnableDifonifmode40 ==
  mc : Inport
  on_off : Inport  Port=2
  Constant7 : Constant  SampleTime=Ts
  Constant8 : Constant  Value=0  SampleTime=Ts
  If Action Subsystem7 : Subsystem  TreatAsAtomicUnit=on
  If Action Subsystem8 : Subsystem  TreatAsAtomicUnit=on
  If5 : If  SampleTime=Ts
  Logical Operator : Logic  AllPortsSameDT=off  OutDataTypeStr=boolean  SampleTime=Ts
  Merge3 : Merge
  enable detection : Outport
  wires:
  mc/1 -> If5/1
  If Action Subsystem8/1(Out1) -> Merge3/2
  If5/2 -> If Action Subsystem8/Action
  If5/1 -> If Action Subsystem7/Action
  Constant8/1 -> If Action Subsystem8/1(In1)
  Constant7/1 -> If Action Subsystem7/1(In1)
  If Action Subsystem7/1(Out1) -> Merge3/1
  Logical Operator/1 -> enable detection/1
  Merge3/1 -> Logical Operator/1
  on_off/1 -> Logical Operator/2

== system controller_observer_AllAgents_v5/Controller/EnableDifonifmode41 ==
  mc : Inport
  on_off : Inport  Port=2
  Constant7 : Constant  SampleTime=Ts
  Constant8 : Constant  Value=0  SampleTime=Ts
  If Action Subsystem7 : Subsystem  TreatAsAtomicUnit=on
  If Action Subsystem8 : Subsystem  TreatAsAtomicUnit=on
  If5 : If  SampleTime=Ts
  Logical Operator : Logic  AllPortsSameDT=off  OutDataTypeStr=boolean  SampleTime=Ts
  Merge3 : Merge
  enable detection : Outport
  wires:
  mc/1 -> If5/1
  If Action Subsystem8/1(Out1) -> Merge3/2
  If5/2 -> If Action Subsystem8/Action
  If5/1 -> If Action Subsystem7/Action
  Constant8/1 -> If Action Subsystem8/1(In1)
  Constant7/1 -> If Action Subsystem7/1(In1)
  If Action Subsystem7/1(Out1) -> Merge3/1
  Logical Operator/1 -> enable detection/1
  Merge3/1 -> Logical Operator/1
  on_off/1 -> Logical Operator/2

== system controller_observer_AllAgents_v5/Controller/EnableDifonifmode42 ==
  mc : Inport
  on_off : Inport  Port=2
  Constant7 : Constant  SampleTime=Ts
  Constant8 : Constant  Value=0  SampleTime=Ts
  If Action Subsystem7 : Subsystem  TreatAsAtomicUnit=on
  If Action Subsystem8 : Subsystem  TreatAsAtomicUnit=on
  If5 : If  SampleTime=Ts
  Logical Operator : Logic  AllPortsSameDT=off  OutDataTypeStr=boolean  SampleTime=Ts
  Merge3 : Merge
  enable detection : Outport
  wires:
  mc/1 -> If5/1
  If Action Subsystem8/1(Out1) -> Merge3/2
  If5/2 -> If Action Subsystem8/Action
  If5/1 -> If Action Subsystem7/Action
  Constant8/1 -> If Action Subsystem8/1(In1)
  Constant7/1 -> If Action Subsystem7/1(In1)
  If Action Subsystem7/1(Out1) -> Merge3/1
  Logical Operator/1 -> enable detection/1
  Merge3/1 -> Logical Operator/1
  on_off/1 -> Logical Operator/2

== system controller_observer_AllAgents_v5/Controller/EnableDifonifmode43 ==
  mc : Inport
  on_off : Inport  Port=2
  Constant7 : Constant  SampleTime=Ts
  Constant8 : Constant  Value=0  SampleTime=Ts
  If Action Subsystem7 : Subsystem  TreatAsAtomicUnit=on
  If Action Subsystem8 : Subsystem  TreatAsAtomicUnit=on
  If5 : If  SampleTime=Ts
  Logical Operator : Logic  AllPortsSameDT=off  OutDataTypeStr=boolean  SampleTime=Ts
  Merge3 : Merge
  enable detection : Outport
  wires:
  mc/1 -> If5/1
  If Action Subsystem8/1(Out1) -> Merge3/2
  If5/2 -> If Action Subsystem8/Action
  If5/1 -> If Action Subsystem7/Action
  Constant8/1 -> If Action Subsystem8/1(In1)
  Constant7/1 -> If Action Subsystem7/1(In1)
  If Action Subsystem7/1(Out1) -> Merge3/1
  Logical Operator/1 -> enable detection/1
  Merge3/1 -> Logical Operator/1
  on_off/1 -> Logical Operator/2

== system controller_observer_AllAgents_v5/Controller/EnableDifonifmode44 ==
  mc : Inport
  on_off : Inport  Port=2
  Constant7 : Constant  SampleTime=Ts
  Constant8 : Constant  Value=0  SampleTime=Ts
  If Action Subsystem7 : Subsystem  TreatAsAtomicUnit=on
  If Action Subsystem8 : Subsystem  TreatAsAtomicUnit=on
  If5 : If  SampleTime=Ts
  Logical Operator : Logic  AllPortsSameDT=off  OutDataTypeStr=boolean  SampleTime=Ts
  Merge3 : Merge
  enable detection : Outport
  wires:
  mc/1 -> If5/1
  If Action Subsystem8/1(Out1) -> Merge3/2
  If5/2 -> If Action Subsystem8/Action
  If5/1 -> If Action Subsystem7/Action
  Constant8/1 -> If Action Subsystem8/1(In1)
  Constant7/1 -> If Action Subsystem7/1(In1)
  If Action Subsystem7/1(Out1) -> Merge3/1
  Logical Operator/1 -> enable detection/1
  Merge3/1 -> Logical Operator/1
  on_off/1 -> Logical Operator/2

== system controller_observer_AllAgents_v5/Controller/EnableDifonifmode45 ==
  mc : Inport
  on_off : Inport  Port=2
  Constant7 : Constant  SampleTime=Ts
  Constant8 : Constant  Value=0  SampleTime=Ts
  If Action Subsystem7 : Subsystem  TreatAsAtomicUnit=on
  If Action Subsystem8 : Subsystem  TreatAsAtomicUnit=on
  If5 : If  SampleTime=Ts
  Logical Operator : Logic  AllPortsSameDT=off  OutDataTypeStr=boolean  SampleTime=Ts
  Merge3 : Merge
  enable detection : Outport
  wires:
  mc/1 -> If5/1
  If Action Subsystem8/1(Out1) -> Merge3/2
  If5/2 -> If Action Subsystem8/Action
  If5/1 -> If Action Subsystem7/Action
  Constant8/1 -> If Action Subsystem8/1(In1)
  Constant7/1 -> If Action Subsystem7/1(In1)
  If Action Subsystem7/1(Out1) -> Merge3/1
  Logical Operator/1 -> enable detection/1
  Merge3/1 -> Logical Operator/1
  on_off/1 -> Logical Operator/2
